simulink model "RC_Demo_CAN_Host"  (R2019b)
settings: Solver=ode45  StartTime=0.0  StopTime=inf  RelTol=1e-3  EnableMultiTasking=on
undefined symbols (not defined in the file): canChannel
libraries: canmsglib x6, canlib x5
settings Model: Creator=The MathWorks, Inc.  LastModifiedBy=bhisma
settings SolverCC: InsertRTBMode=Auto
settings DataIOCC: LimitDataPoints=on  SaveFormat=Array  ReturnWorkspaceOutputs=off  SignalLoggingName=sigsOut
settings OptimizationCC: ExpressionDepthLimit=2147483647  InitFltsAndDblsToZero=on  LifeSpan=inf
settings DebuggingCC: SaveWithParameterizedLinksMsg=none  UnderspecifiedInitializationDetection=Classic  MergeDetectMultiDrivingBlocksExec=none  CheckExecutionContextPreStartOutputMsg=on  CheckExecutionContextRuntimeOutputMsg=on  SignalResolutionControl=TryResolveAllWithWarning  MultiTaskDSMMsg=warning  MultiTaskCondExecSysMsg=none  FcnCallInpInsideContextMsg=warning  StateNameClashWarn=warning  SFUnexpectedBacktrackingDiag=warning  SFNoUnconditionalDefaultTransitionDiag=warning  SFExecutionAtInitializationDiag=none  ArithmeticOperatorsInVariantConditions=warning
settings HardwareCC: ProdBitPerPointer=32  ProdBitPerSizeT=32  ProdBitPerPtrDiffT=32  ProdLargestAtomicFloat=None  ProdIntDivRoundTo=Undefined  ProdEndianess=Unspecified  ProdWordSize=32  ProdHWDeviceType=Specified
settings ModelReferenceCC: PropagateSignalLabelsOutOfModel=off
settings SFSimCC: SimUseLocalCustomCode=off  CustomCodeUndefinedFunction=UseInterfaceOnly
settings RTWCC: RTWUseLocalCustomCode=off
settings CodeAppCC: StateflowObjectComments=on
settings GRTTargetCC: TargetFcnLib=ansi_tfl_tmw.mat  TargetLangStandard=C89/C90 (ANSI)  CombineOutputUpdateFcns=off  GRTInterface=on
settings ConfigComp: CovMetricSettings=dw  CovHtmlReporting=on  CovSaveCumulativeToWorkspaceVar=on  CovSaveSingleToWorkspaceVar=on  CovExternalEMLEnable=off

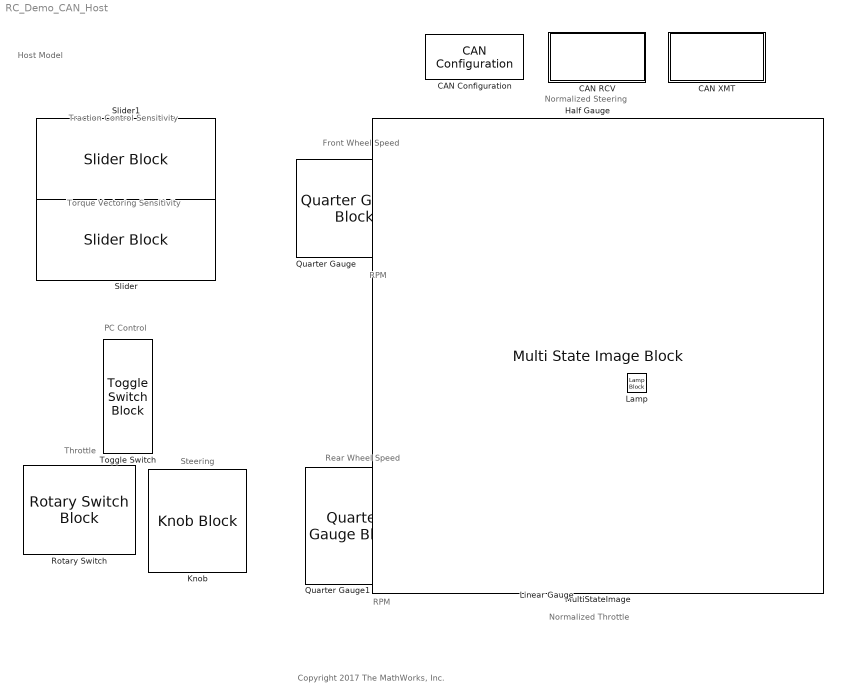
== system RC_Demo_CAN_Host ==
  note: Front Wheel Speed
  note: Host Model
  note: Copyright 2017 The MathWorks, Inc.
  note: Rear Wheel Speed
  note: RPM
  note: RPM
  note: PC Control
  note: Traction Control Sensitivity
  note: Torque Vectoring Sensitivity
  note: Steering
  note: Throttle
  note: Normalized Steering
  note: Normalized Throttle
  CAN Configuration : canlib/CAN Configuration  BlockHandle=5.8817  DeviceMenu=MathWorks Virtual 1 (Channel 1)  Device=Vector CANcaseXL 1 (Channel 2)  ObjConstructor=canChannel('Vector','CANcaseXL 1',2)  BusSpeed=500000  EnableBitParameters=off  SJW=1  TSEG1=4  TSEG2=3  NSamples=1  AckMode=Normal
      settings: SourceProductName=Vehicle Network Toolbox  SourceProductBaseCode=VN
  CAN RCV : Subsystem
  CAN XMT : Subsystem
  Half Gauge : SemiCircularGaugeBlock  ScaleMin=-1  ScaleMax=1
  Knob : KnobBlock  ScaleMin=-1  ScaleMax=1
  Lamp : LampBlock  LabelPosition=Hide
  Linear Gauge : LinearGaugeBlock  ScaleMin=-1  ScaleMax=1
  MultiStateImage : MultiStateImageBlock  LabelPosition=Hide
  Quarter Gauge : QuarterGaugeBlock  ScaleMax=1000
  Quarter Gauge1 : QuarterGaugeBlock  ScaleMax=1000
  Rotary Switch : RotarySwitchBlock  LabelPosition=Hide
      settings: Values={{'Reverse', '0', 'Forward'}, [-0.3 0 0.3]}
  Slider : SliderBlock  ScaleMax=1
  Slider1 : SliderBlock  ScaleMax=1
  Toggle Switch : ToggleSwitchBlock  LabelPosition=Hide
      settings: Values={{'Off', 'On'}, [0 1]}

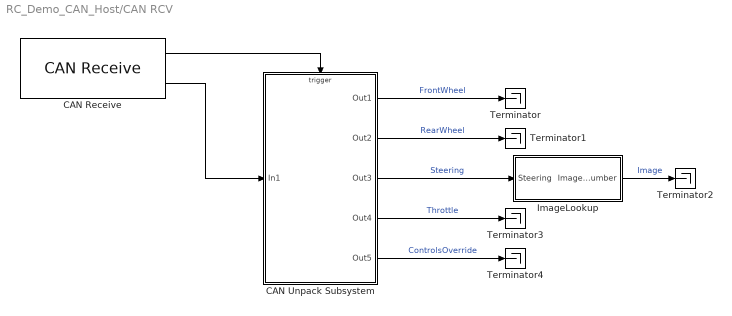
== system RC_Demo_CAN_Host/CAN RCV ==
  CAN Receive : canlib/CAN Receive  BlockHandle=7.0039  DeviceMenu=MathWorks Virtual 1 (Channel 1)  Device=Vector CANcaseXL 1 (Channel 2)  ObjConstructor=canChannel('Vector','CANcaseXL 1',2)  EnableStandardIDsFilter=off  StandardIDs=[48 33 65]  EnableExtendedIDsFilter=off  ExtendedIDs=0  SampleTime=0.2  MsgsPerTimestep=all  StdIDsCombo=Allow only  ExtIDsCombo=Allow all  InternalFilterUpdateStatus=on
      settings: SourceProductName=Vehicle Network Toolbox  SourceProductBaseCode=VN
  CAN Unpack Subsystem : Subsystem  TreatAsAtomicUnit=on
  ImageLookup : Subsystem
  Terminator{,1..4} (5 alike) : Terminator
  wires:
  CAN Unpack Subsystem/4(Out4) -> Terminator3/1  "Throttle"
  CAN Unpack Subsystem/3(Out3) -> ImageLookup/1(Steering)  "Steering"
  CAN Receive/1 -> CAN Unpack Subsystem/Trigger
  CAN Unpack Subsystem/2(Out2) -> Terminator1/1  "RearWheel"
  CAN Unpack Subsystem/1(Out1) -> Terminator/1  "FrontWheel"
  CAN Receive/2 -> CAN Unpack Subsystem/1(In1)
  CAN Unpack Subsystem/5(Out5) -> Terminator4/1  "ControlsOverride"
  ImageLookup/1(ImageNumber) -> Terminator2/1  "Image"

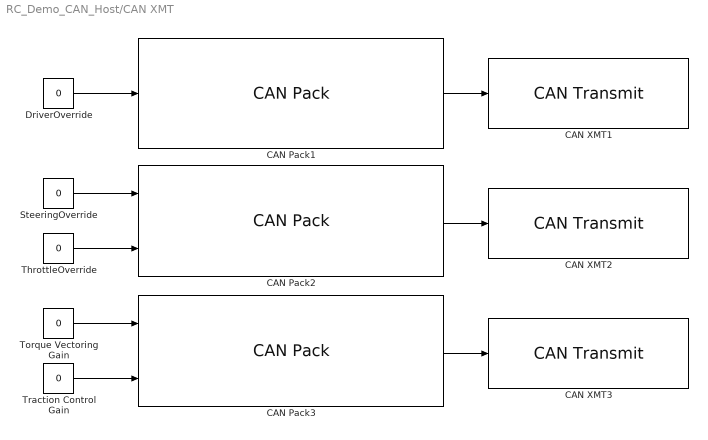
== system RC_Demo_CAN_Host/CAN XMT ==
  CAN Pack1 : canmsglib/CAN Pack  CANdbFile=C:\Users\josea\Desktop\C2000\CAN\Vector\RCDemo.dbc  MsgList=DriverOverrideToCar  MsgName=DriverOverrideToCar  MsgIDType=Standard (11-bit identifier)  MsgIdentifier=64  MsgLength=1  SignalInfo=DriverOverride$0$8$1$1$1$0$1$0$0$1$#  NSignals=1  StartBits=str2num('[int32(0)]')  SignalSizes=str2num('[int32(8)]')  ByteOrders=str2num('[int32(1)]')  DataTypes=str2num('[int32(1)]')  MultiplexTypes=str2num('[int32(1)]')  MultiplexValues=str2num('[double(0)]')  Factors=str2num('[double(1)]')  Offsets=str2num('[double(0)]')  Minimums=str2num('[double(0)]')  Maximums=str2num('[double(1)]')  Remote=off  ApplyMinMax=off
      settings: SourceProductName=Simulink Real-Time  DataFormat=CANdb specified signals
  CAN Pack2 : canmsglib/CAN Pack  CANdbFile=C:\Users\josea\Desktop\C2000\CAN\Vector\RCDemo.dbc  MsgList=DriverControlsToCar  MsgName=DriverControlsToCar  MsgIDType=Standard (11-bit identifier)  MsgIdentifier=32  MsgLength=8  SignalInfo=SteeringInput$32$32$1$3$1$0$1$0$-3.400000e+38$3.400000e+38$#ThrottleInput$0$32$1$3$1$0$1$0$-3.400000e+38$3.400000e+38$#  NSignals=2  StartBits=str2num('[int32(32);int32(0)]')  SignalSizes=str2num('[int32(32);int32(32)]')  ByteOrders=str2num('[int32(1);int32(1)]')  DataTypes=str2num('[int32(3);int32(3)]')  MultiplexTypes=str2num('[int32(1);int32(1)]')  MultiplexValues=str2num('[double(0);double(0)]')  Factors=str2num('[double(1);double(1)]')  Offsets=str2num('[double(0);double(0)]')  Minimums=str2num('[double(-3.400000e+38);double(-3.400000e+38)]')  Maximums=str2num('[double(3.400000e+38);double(3.400000e+38)]')  Remote=off  ApplyMinMax=off
      settings: SourceProductName=Simulink Real-Time  DataFormat=CANdb specified signals
  CAN Pack3 : canmsglib/CAN Pack  CANdbFile=C:\Users\josea\Desktop\C2000\CAN\Vector\RCDemo.dbc  MsgList=DynamicsControllerGains  MsgName=DynamicsControllerGains  MsgIDType=Standard (11-bit identifier)  MsgIdentifier=16  MsgLength=8  SignalInfo=TorqueVectoringGain$0$32$1$3$1$0$1$0$0$1$#TractionControlGain$32$32$1$3$1$0$1$0$0$1$#  NSignals=2  StartBits=str2num('[int32(0);int32(32)]')  SignalSizes=str2num('[int32(32);int32(32)]')  ByteOrders=str2num('[int32(1);int32(1)]')  DataTypes=str2num('[int32(3);int32(3)]')  MultiplexTypes=str2num('[int32(1);int32(1)]')  MultiplexValues=str2num('[double(0);double(0)]')  Factors=str2num('[double(1);double(1)]')  Offsets=str2num('[double(0);double(0)]')  Minimums=str2num('[double(0);double(0)]')  Maximums=str2num('[double(1);double(1)]')  Remote=off  ApplyMinMax=off
      settings: SourceProductName=Simulink Real-Time  DataFormat=CANdb specified signals
  CAN XMT1 : canlib/CAN Transmit  BlockHandle=54.0027  DeviceMenu=MathWorks Virtual 1 (Channel 1)  Device=Vector CANcaseXL 1 (Channel 2)  ObjConstructor=canChannel('Vector','CANcaseXL 1',2)  EnablePeriodicTransmit=on  MessagePeriod=0.025
      settings: SourceProductName=Vehicle Network Toolbox  SourceProductBaseCode=VN
  CAN XMT2 : canlib/CAN Transmit  BlockHandle=55.0032  DeviceMenu=MathWorks Virtual 1 (Channel 1)  Device=Vector CANcaseXL 1 (Channel 2)  ObjConstructor=canChannel('Vector','CANcaseXL 1',2)  EnablePeriodicTransmit=on  MessagePeriod=0.025
      settings: SourceProductName=Vehicle Network Toolbox  SourceProductBaseCode=VN
  CAN XMT3 : canlib/CAN Transmit  BlockHandle=56.0029  DeviceMenu=MathWorks Virtual 1 (Channel 1)  Device=Vector CANcaseXL 1 (Channel 2)  ObjConstructor=canChannel('Vector','CANcaseXL 1',2)  EnablePeriodicTransmit=on  MessagePeriod=0.025
      settings: SourceProductName=Vehicle Network Toolbox  SourceProductBaseCode=VN
  DriverOverride : Constant  Value=0
  SteeringOverride : Constant  Value=0
  ThrottleOverride : Constant  Value=0
  Torque Vectoring Gain : Constant  Value=0
  Traction Control Gain : Constant  Value=0
  wires:
  DriverOverride/1 -> CAN Pack1/1
  CAN Pack1/1 -> CAN XMT1/1
  CAN Pack2/1 -> CAN XMT2/1
  SteeringOverride/1 -> CAN Pack2/1
  ThrottleOverride/1 -> CAN Pack2/2
  CAN Pack3/1 -> CAN XMT3/1
  Traction Control Gain/1 -> CAN Pack3/2
  Torque Vectoring Gain/1 -> CAN Pack3/1

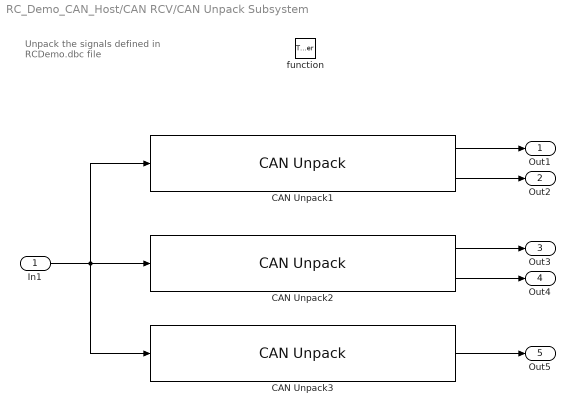
== system RC_Demo_CAN_Host/CAN RCV/CAN Unpack Subsystem ==
  note: Unpack the signals
      | defined in RCDemo.dbc file
  In1 : Inport
  function : TriggerPort  TriggerType=function-call  VariantControl=Variant
  CAN Unpack1 : canmsglib/CAN Unpack  CANdbFile=C:\Users\josea\Desktop\C2000\CAN\Vector\RCDemo.dbc  MsgList=WheelSpeeds  MsgName=WheelSpeeds  MsgIDType=Standard (11-bit identifier)  MsgIdentifier=48  MsgLength=8  SignalInfo=FrontWheelSpeed$24$32$2$3$1$0$1$0$-3.400000e+38$3.400000e+38$#RearWheelSpeed$56$32$2$3$1$0$1$0$-3.400000e+38$3.400000e+38$#  NSignals=2  IDPort=off  TimestampPort=off  ErrorPort=off  RemotePort=off  LengthPort=off  StatusPort=off  StartBits=str2num('[int32(24);int32(56)]')  SignalSizes=str2num('[int32(32);int32(32)]')  ByteOrders=str2num('[int32(2);int32(2)]')  DataTypes=str2num('[int32(3);int32(3)]')  MultiplexTypes=str2num('[int32(1);int32(1)]')  MultiplexValues=str2num('[double(0);double(0)]')  Factors=str2num('[double(1);double(1)]')  Offsets=str2num('[double(0);double(0)]')  Minimums=str2num('[double(-3.400000e+38);double(-3.400000e+38)]')  Maximums=str2num('[double(3.400000e+38);double(3.400000e+38)]')  ApplyMinMax=off
      settings: SourceProductName=Simulink Real-Time  DataFormat=CANdb specified signals
  CAN Unpack2 : canmsglib/CAN Unpack  CANdbFile=C:\Users\josea\Desktop\C2000\CAN\Vector\RCDemo.dbc  MsgList=DriverControlsFromCar  MsgName=DriverControlsFromCar  MsgIDType=Standard (11-bit identifier)  MsgIdentifier=33  MsgLength=8  SignalInfo=SteeringInput$32$32$1$3$1$0$1$0$-3.400000e+38$3.400000e+38$#ThrottleInput$0$32$1$3$1$0$1$0$-3.400000e+38$3.400000e+38$#  NSignals=2  IDPort=off  TimestampPort=off  ErrorPort=off  RemotePort=off  LengthPort=off  StatusPort=off  StartBits=str2num('[int32(32);int32(0)]')  SignalSizes=str2num('[int32(32);int32(32)]')  ByteOrders=str2num('[int32(1);int32(1)]')  DataTypes=str2num('[int32(3);int32(3)]')  MultiplexTypes=str2num('[int32(1);int32(1)]')  MultiplexValues=str2num('[double(0);double(0)]')  Factors=str2num('[double(1);double(1)]')  Offsets=str2num('[double(0);double(0)]')  Minimums=str2num('[double(-3.400000e+38);double(-3.400000e+38)]')  Maximums=str2num('[double(3.400000e+38);double(3.400000e+38)]')  ApplyMinMax=off
      settings: SourceProductName=Simulink Real-Time  DataFormat=CANdb specified signals
  CAN Unpack3 : canmsglib/CAN Unpack  CANdbFile=C:\Users\josea\Desktop\C2000\CAN\Vector\RCDemo.dbc  MsgList=DriverOverrideFromCar  MsgName=DriverOverrideFromCar  MsgIDType=Standard (11-bit identifier)  MsgIdentifier=65  MsgLength=1  SignalInfo=DriverOverride$0$8$2$1$1$0$1$0$0$1$#  NSignals=1  IDPort=off  TimestampPort=off  ErrorPort=off  RemotePort=off  LengthPort=off  StatusPort=off  StartBits=str2num('[int32(0)]')  SignalSizes=str2num('[int32(8)]')  ByteOrders=str2num('[int32(2)]')  DataTypes=str2num('[int32(1)]')  MultiplexTypes=str2num('[int32(1)]')  MultiplexValues=str2num('[double(0)]')  Factors=str2num('[double(1)]')  Offsets=str2num('[double(0)]')  Minimums=str2num('[double(0)]')  Maximums=str2num('[double(1)]')  ApplyMinMax=off
      settings: SourceProductName=Simulink Real-Time  DataFormat=CANdb specified signals
  Out1 : Outport
  Out2 : Outport  Port=2
  Out3 : Outport  Port=3
  Out4 : Outport  Port=4
  Out5 : Outport  Port=5
  wires:
  CAN Unpack1/1 -> Out1/1
  In1/1 -> CAN Unpack1/1, CAN Unpack3/1, CAN Unpack2/1
  CAN Unpack1/2 -> Out2/1
  CAN Unpack2/1 -> Out3/1
  CAN Unpack2/2 -> Out4/1
  CAN Unpack3/1 -> Out5/1

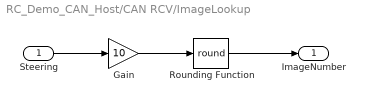
== system RC_Demo_CAN_Host/CAN RCV/ImageLookup ==
  Steering : Inport
  Gain : Gain  Gain=10
  Rounding Function : Rounding  Operator=round
  ImageNumber : Outport
  wires:
  Steering/1 -> Gain/1
  Gain/1 -> Rounding Function/1
  Rounding Function/1 -> ImageNumber/1
